simulink model "doa_debug_diego"  (R2013b) (continued)
== system doa_debug_diego == (continued)
      settings: AttributesFormatString=Latency = %<latency>
      mask Display:
      | port_label('input', 1, 'd');
      | port_label('output', 1, 'q');
      | disp(['Z^-', num2str(latency)]);
  pipeline65 : Subsystem  AncestorBlock=casper_library_delays/pipeline  mask(pipeline){latency=2}
      mask help:
      | An explicitly laid-out delay line to avoid mapping to SRL16s
      | for use in pipelining to help achieve timing closure.
      mask init:
      | pipeline_init(gcb,'latency',latency);
      settings: AttributesFormatString=Latency = %<latency>
      mask Display:
      | port_label('input', 1, 'd');
      | port_label('output', 1, 'q');
      | disp(['Z^-', num2str(latency)]);
  pipeline66 : Subsystem  AncestorBlock=casper_library_delays/pipeline  mask(pipeline){latency=2}
      mask help:
      | An explicitly laid-out delay line to avoid mapping to SRL16s
      | for use in pipelining to help achieve timing closure.
      mask init:
      | pipeline_init(gcb,'latency',latency);
      settings: AttributesFormatString=Latency = %<latency>
      mask Display:
      | port_label('input', 1, 'd');
      | port_label('output', 1, 'q');
      | disp(['Z^-', num2str(latency)]);
  pipeline67 : Subsystem  AncestorBlock=casper_library_delays/pipeline  mask(pipeline){latency=1}
      mask help:
      | An explicitly laid-out delay line to avoid mapping to SRL16s
      | for use in pipelining to help achieve timing closure.
      mask init:
      | pipeline_init(gcb,'latency',latency);
      settings: AttributesFormatString=Latency = %<latency>
      mask Display:
      | port_label('input', 1, 'd');
      | port_label('output', 1, 'q');
      | disp(['Z^-', num2str(latency)]);
  pipeline68 : Subsystem  AncestorBlock=casper_library_delays/pipeline  mask(pipeline){latency=1}
      mask help:
      | An explicitly laid-out delay line to avoid mapping to SRL16s
      | for use in pipelining to help achieve timing closure.
      mask init:
      | pipeline_init(gcb,'latency',latency);
      settings: AttributesFormatString=Latency = %<latency>
      mask Display:
      | port_label('input', 1, 'd');
      | port_label('output', 1, 'q');
      | disp(['Z^-', num2str(latency)]);
  pipeline69 : Subsystem  AncestorBlock=casper_library_delays/pipeline  mask(pipeline){latency=2}
      mask help:
      | An explicitly laid-out delay line to avoid mapping to SRL16s
      | for use in pipelining to help achieve timing closure.
      mask init:
      | pipeline_init(gcb,'latency',latency);
      settings: AttributesFormatString=Latency = %<latency>
      mask Display:
      | port_label('input', 1, 'd');
      | port_label('output', 1, 'q');
      | disp(['Z^-', num2str(latency)]);
  pipeline70 : Subsystem  AncestorBlock=casper_library_delays/pipeline  mask(pipeline){latency=2}
      mask help:
      | An explicitly laid-out delay line to avoid mapping to SRL16s
      | for use in pipelining to help achieve timing closure.
      mask init:
      | pipeline_init(gcb,'latency',latency);
      settings: AttributesFormatString=Latency = %<latency>
      mask Display:
      | port_label('input', 1, 'd');
      | port_label('output', 1, 'q');
      | disp(['Z^-', num2str(latency)]);
  pipeline71 : Subsystem  AncestorBlock=casper_library_delays/pipeline  mask(pipeline){latency=1}
      mask help:
      | An explicitly laid-out delay line to avoid mapping to SRL16s
      | for use in pipelining to help achieve timing closure.
      mask init:
      | pipeline_init(gcb,'latency',latency);
      settings: AttributesFormatString=Latency = %<latency>
      mask Display:
      | port_label('input', 1, 'd');
      | port_label('output', 1, 'q');
      | disp(['Z^-', num2str(latency)]);
  pipeline72 : Subsystem  AncestorBlock=casper_library_delays/pipeline  mask(pipeline){latency=5}
      mask help:
      | An explicitly laid-out delay line to avoid mapping to SRL16s
      | for use in pipelining to help achieve timing closure.
      mask init:
      | pipeline_init(gcb,'latency',latency);
      settings: AttributesFormatString=Latency = %<latency>
      mask Display:
      | port_label('input', 1, 'd');
      | port_label('output', 1, 'q');
      | disp(['Z^-', num2str(latency)]);
  pipeline73 : Subsystem  AncestorBlock=casper_library_delays/pipeline  mask(pipeline){latency=1}
      mask help:
      | An explicitly laid-out delay line to avoid mapping to SRL16s
      | for use in pipelining to help achieve timing closure.
      mask init:
      | pipeline_init(gcb,'latency',latency);
      settings: AttributesFormatString=Latency = %<latency>
      mask Display:
      | port_label('input', 1, 'd');
      | port_label('output', 1, 'q');
      | disp(['Z^-', num2str(latency)]);
  pipeline74 : Subsystem  AncestorBlock=casper_library_delays/pipeline  mask(pipeline){latency=2}
      mask help:
      | An explicitly laid-out delay line to avoid mapping to SRL16s
      | for use in pipelining to help achieve timing closure.
      mask init:
      | pipeline_init(gcb,'latency',latency);
      settings: AttributesFormatString=Latency = %<latency>
      mask Display:
      | port_label('input', 1, 'd');
      | port_label('output', 1, 'q');
      | disp(['Z^-', num2str(latency)]);
  pipeline75 : Subsystem  AncestorBlock=casper_library_delays/pipeline  mask(pipeline){latency=2}
      mask help:
      | An explicitly laid-out delay line to avoid mapping to SRL16s
      | for use in pipelining to help achieve timing closure.
      mask init:
      | pipeline_init(gcb,'latency',latency);
      settings: AttributesFormatString=Latency = %<latency>
      mask Display:
      | port_label('input', 1, 'd');
      | port_label('output', 1, 'q');
      | disp(['Z^-', num2str(latency)]);
  pipeline76 : Subsystem  AncestorBlock=casper_library_delays/pipeline  mask(pipeline){latency=1}
      mask help:
      | An explicitly laid-out delay line to avoid mapping to SRL16s
      | for use in pipelining to help achieve timing closure.
      mask init:
      | pipeline_init(gcb,'latency',latency);
      settings: AttributesFormatString=Latency = %<latency>
      mask Display:
      | port_label('input', 1, 'd');
      | port_label('output', 1, 'q');
      | disp(['Z^-', num2str(latency)]);
  pipeline77 : Subsystem  AncestorBlock=casper_library_delays/pipeline  mask(pipeline){latency=1}
      mask help:
      | An explicitly laid-out delay line to avoid mapping to SRL16s
      | for use in pipelining to help achieve timing closure.
      mask init:
      | pipeline_init(gcb,'latency',latency);
      settings: AttributesFormatString=Latency = %<latency>
      mask Display:
      | port_label('input', 1, 'd');
      | port_label('output', 1, 'q');
      | disp(['Z^-', num2str(latency)]);
  pipeline78 : Subsystem  AncestorBlock=casper_library_delays/pipeline  mask(pipeline){latency=1}
      mask help:
      | An explicitly laid-out delay line to avoid mapping to SRL16s
      | for use in pipelining to help achieve timing closure.
      mask init:
      | pipeline_init(gcb,'latency',latency);
      settings: AttributesFormatString=Latency = %<latency>
      mask Display:
      | port_label('input', 1, 'd');
      | port_label('output', 1, 'q');
      | disp(['Z^-', num2str(latency)]);
  pipeline79 : Subsystem  AncestorBlock=casper_library_delays/pipeline  mask(pipeline){latency=2}
      mask help:
      | An explicitly laid-out delay line to avoid mapping to SRL16s
      | for use in pipelining to help achieve timing closure.
      mask init:
      | pipeline_init(gcb,'latency',latency);
      settings: AttributesFormatString=Latency = %<latency>
      mask Display:
      | port_label('input', 1, 'd');
      | port_label('output', 1, 'q');
      | disp(['Z^-', num2str(latency)]);
  pipeline8 : Subsystem  AncestorBlock=casper_library_delays/pipeline  mask(pipeline){latency=9}
      mask help:
      | An explicitly laid-out delay line to avoid mapping to SRL16s
      | for use in pipelining to help achieve timing closure.
      mask init:
      | pipeline_init(gcb,'latency',latency);
      settings: AttributesFormatString=Latency = %<latency>
      mask Display:
      | port_label('input', 1, 'd');
      | port_label('output', 1, 'q');
      | disp(['Z^-', num2str(latency)]);
  pipeline80 : Subsystem  AncestorBlock=casper_library_delays/pipeline  mask(pipeline){latency=2}
      mask help:
      | An explicitly laid-out delay line to avoid mapping to SRL16s
      | for use in pipelining to help achieve timing closure.
      mask init:
      | pipeline_init(gcb,'latency',latency);
      settings: AttributesFormatString=Latency = %<latency>
      mask Display:
      | port_label('input', 1, 'd');
      | port_label('output', 1, 'q');
      | disp(['Z^-', num2str(latency)]);
  pipeline81 : Subsystem  AncestorBlock=casper_library_delays/pipeline  mask(pipeline){latency=1}
      mask help:
      | An explicitly laid-out delay line to avoid mapping to SRL16s
      | for use in pipelining to help achieve timing closure.
      mask init:
      | pipeline_init(gcb,'latency',latency);
      settings: AttributesFormatString=Latency = %<latency>
      mask Display:
      | port_label('input', 1, 'd');
      | port_label('output', 1, 'q');
      | disp(['Z^-', num2str(latency)]);
  pipeline82 : Subsystem  AncestorBlock=casper_library_delays/pipeline  mask(pipeline){latency=1}
      mask help:
      | An explicitly laid-out delay line to avoid mapping to SRL16s
      | for use in pipelining to help achieve timing closure.
      mask init:
      | pipeline_init(gcb,'latency',latency);
      settings: AttributesFormatString=Latency = %<latency>
      mask Display:
      | port_label('input', 1, 'd');
      | port_label('output', 1, 'q');
      | disp(['Z^-', num2str(latency)]);
  pipeline83 : Subsystem  AncestorBlock=casper_library_delays/pipeline  mask(pipeline){latency=2}
      mask help:
      | An explicitly laid-out delay line to avoid mapping to SRL16s
      | for use in pipelining to help achieve timing closure.
      mask init:
      | pipeline_init(gcb,'latency',latency);
      settings: AttributesFormatString=Latency = %<latency>
      mask Display:
      | port_label('input', 1, 'd');
      | port_label('output', 1, 'q');
      | disp(['Z^-', num2str(latency)]);
  pipeline84 : Subsystem  AncestorBlock=casper_library_delays/pipeline  mask(pipeline){latency=2}
      mask help:
      | An explicitly laid-out delay line to avoid mapping to SRL16s
      | for use in pipelining to help achieve timing closure.
      mask init:
      | pipeline_init(gcb,'latency',latency);
      settings: AttributesFormatString=Latency = %<latency>
      mask Display:
      | port_label('input', 1, 'd');
      | port_label('output', 1, 'q');
      | disp(['Z^-', num2str(latency)]);
  pipeline85 : Subsystem  AncestorBlock=casper_library_delays/pipeline  mask(pipeline){latency=1}
      mask help:
      | An explicitly laid-out delay line to avoid mapping to SRL16s
      | for use in pipelining to help achieve timing closure.
      mask init:
      | pipeline_init(gcb,'latency',latency);
      settings: AttributesFormatString=Latency = %<latency>
      mask Display:
      | port_label('input', 1, 'd');
      | port_label('output', 1, 'q');
      | disp(['Z^-', num2str(latency)]);
  pipeline86 : Subsystem  AncestorBlock=casper_library_delays/pipeline  mask(pipeline){latency=1}
      mask help:
      | An explicitly laid-out delay line to avoid mapping to SRL16s
      | for use in pipelining to help achieve timing closure.
      mask init:
      | pipeline_init(gcb,'latency',latency);
      settings: AttributesFormatString=Latency = %<latency>
      mask Display:
      | port_label('input', 1, 'd');
      | port_label('output', 1, 'q');
      | disp(['Z^-', num2str(latency)]);
  pipeline87 : Subsystem  AncestorBlock=casper_library_delays/pipeline  mask(pipeline){latency=2}
      mask help:
      | An explicitly laid-out delay line to avoid mapping to SRL16s
      | for use in pipelining to help achieve timing closure.
      mask init:
      | pipeline_init(gcb,'latency',latency);
      settings: AttributesFormatString=Latency = %<latency>
      mask Display:
      | port_label('input', 1, 'd');
      | port_label('output', 1, 'q');
      | disp(['Z^-', num2str(latency)]);
  pipeline88 : Subsystem  AncestorBlock=casper_library_delays/pipeline  mask(pipeline){latency=2}
      mask help:
      | An explicitly laid-out delay line to avoid mapping to SRL16s
      | for use in pipelining to help achieve timing closure.
      mask init:
      | pipeline_init(gcb,'latency',latency);
      settings: AttributesFormatString=Latency = %<latency>
      mask Display:
      | port_label('input', 1, 'd');
      | port_label('output', 1, 'q');
      | disp(['Z^-', num2str(latency)]);
  pipeline89 : Subsystem  AncestorBlock=casper_library_delays/pipeline  mask(pipeline){latency=1}
      mask help:
      | An explicitly laid-out delay line to avoid mapping to SRL16s
      | for use in pipelining to help achieve timing closure.
      mask init:
      | pipeline_init(gcb,'latency',latency);
      settings: AttributesFormatString=Latency = %<latency>
      mask Display:
      | port_label('input', 1, 'd');
      | port_label('output', 1, 'q');
      | disp(['Z^-', num2str(latency)]);
  pipeline90 : Subsystem  AncestorBlock=casper_library_delays/pipeline  mask(pipeline){latency=5}
      mask help:
      | An explicitly laid-out delay line to avoid mapping to SRL16s
      | for use in pipelining to help achieve timing closure.
      mask init:
      | pipeline_init(gcb,'latency',latency);
      settings: AttributesFormatString=Latency = %<latency>
      mask Display:
      | port_label('input', 1, 'd');
      | port_label('output', 1, 'q');
      | disp(['Z^-', num2str(latency)]);
  pipeline91 : Subsystem  AncestorBlock=casper_library_delays/pipeline  mask(pipeline){latency=1}
      mask help:
      | An explicitly laid-out delay line to avoid mapping to SRL16s
      | for use in pipelining to help achieve timing closure.
      mask init:
      | pipeline_init(gcb,'latency',latency);
      settings: AttributesFormatString=Latency = %<latency>
      mask Display:
      | port_label('input', 1, 'd');
      | port_label('output', 1, 'q');
      | disp(['Z^-', num2str(latency)]);
  pipeline92 : Subsystem  AncestorBlock=casper_library_delays/pipeline  mask(pipeline){latency=2}
      mask help:
      | An explicitly laid-out delay line to avoid mapping to SRL16s
      | for use in pipelining to help achieve timing closure.
      mask init:
      | pipeline_init(gcb,'latency',latency);
      settings: AttributesFormatString=Latency = %<latency>
      mask Display:
      | port_label('input', 1, 'd');
      | port_label('output', 1, 'q');
      | disp(['Z^-', num2str(latency)]);
  power{,1..7} (8 alike) : casper_library_misc/power  BitWidth=18  add_latency=2  mult_latency=3
  pulse_ext : casper_library_misc/pulse_ext  pulse_len=512
  red0_0 : Subsystem  Tag=xps:bram  AncestorBlock=xps_library/Shared_BRAM  mask{arith_type=Unsigned, addr_width=10, data_width=32, reg_prim_output=off, reg_core_output=off, optimization=Minimum_Area, data_bin_pt=0, init_vals=[0:2^10-1], sample_rate=1}
      mask prompts: arith_type "Output Data Type"; addr_width "Address width"; data_width "Data Width"; reg_prim_output "Register Primitive Output"; reg_core_output "Register Core Output"; data_bin_pt "Data Binary Point"; init_vals "Initial values (simulation only)"; sample_rate "Sample rate"
      mask init:
      | shared_bram_mask;
      mask TypeOptions arith_type: Unsigned | Signed  (2's comp)
      mask TypeOptions data_width: 8 | 16 | 32 | 64 | 128
      mask TypeOptions optimization: Minimum_Area | Low_Power
  red0_1 : Subsystem  Tag=xps:bram  AncestorBlock=xps_library/Shared_BRAM  mask{arith_type=Unsigned, addr_width=10, data_width=32, reg_prim_output=off, reg_core_output=off, optimization=Minimum_Area, data_bin_pt=0, init_vals=[0:2^10-1], sample_rate=1}
      mask prompts: arith_type "Output Data Type"; addr_width "Address width"; data_width "Data Width"; reg_prim_output "Register Primitive Output"; reg_core_output "Register Core Output"; data_bin_pt "Data Binary Point"; init_vals "Initial values (simulation only)"; sample_rate "Sample rate"
      mask init:
      | shared_bram_mask;
      mask TypeOptions arith_type: Unsigned | Signed  (2's comp)
      mask TypeOptions data_width: 8 | 16 | 32 | 64 | 128
      mask TypeOptions optimization: Minimum_Area | Low_Power
  red0_2 : Subsystem  Tag=xps:bram  AncestorBlock=xps_library/Shared_BRAM  mask{arith_type=Unsigned, addr_width=10, data_width=32, reg_prim_output=off, reg_core_output=off, optimization=Minimum_Area, data_bin_pt=0, init_vals=[0:2^10-1], sample_rate=1}
      mask prompts: arith_type "Output Data Type"; addr_width "Address width"; data_width "Data Width"; reg_prim_output "Register Primitive Output"; reg_core_output "Register Core Output"; data_bin_pt "Data Binary Point"; init_vals "Initial values (simulation only)"; sample_rate "Sample rate"
      mask init:
      | shared_bram_mask;
      mask TypeOptions arith_type: Unsigned | Signed  (2's comp)
      mask TypeOptions data_width: 8 | 16 | 32 | 64 | 128
      mask TypeOptions optimization: Minimum_Area | Low_Power
  red0_3 : Subsystem  Tag=xps:bram  AncestorBlock=xps_library/Shared_BRAM  mask{arith_type=Unsigned, addr_width=10, data_width=32, reg_prim_output=off, reg_core_output=off, optimization=Minimum_Area, data_bin_pt=0, init_vals=[0:2^10-1], sample_rate=1}
      mask prompts: arith_type "Output Data Type"; addr_width "Address width"; data_width "Data Width"; reg_prim_output "Register Primitive Output"; reg_core_output "Register Core Output"; data_bin_pt "Data Binary Point"; init_vals "Initial values (simulation only)"; sample_rate "Sample rate"
      mask init:
      | shared_bram_mask;
      mask TypeOptions arith_type: Unsigned | Signed  (2's comp)
      mask TypeOptions data_width: 8 | 16 | 32 | 64 | 128
      mask TypeOptions optimization: Minimum_Area | Low_Power
  red1_0 : Subsystem  Tag=xps:bram  AncestorBlock=xps_library/Shared_BRAM  mask{arith_type=Unsigned, addr_width=10, data_width=32, reg_prim_output=off, reg_core_output=off, optimization=Minimum_Area, data_bin_pt=0, init_vals=[0:2^10-1], sample_rate=1}
      mask prompts: arith_type "Output Data Type"; addr_width "Address width"; data_width "Data Width"; reg_prim_output "Register Primitive Output"; reg_core_output "Register Core Output"; data_bin_pt "Data Binary Point"; init_vals "Initial values (simulation only)"; sample_rate "Sample rate"
      mask init:
      | shared_bram_mask;
      mask TypeOptions arith_type: Unsigned | Signed  (2's comp)
      mask TypeOptions data_width: 8 | 16 | 32 | 64 | 128
      mask TypeOptions optimization: Minimum_Area | Low_Power
  red1_1 : Subsystem  Tag=xps:bram  AncestorBlock=xps_library/Shared_BRAM  mask{arith_type=Unsigned, addr_width=10, data_width=32, reg_prim_output=off, reg_core_output=off, optimization=Minimum_Area, data_bin_pt=0, init_vals=[0:2^10-1], sample_rate=1}
      mask prompts: arith_type "Output Data Type"; addr_width "Address width"; data_width "Data Width"; reg_prim_output "Register Primitive Output"; reg_core_output "Register Core Output"; data_bin_pt "Data Binary Point"; init_vals "Initial values (simulation only)"; sample_rate "Sample rate"
      mask init:
      | shared_bram_mask;
      mask TypeOptions arith_type: Unsigned | Signed  (2's comp)
      mask TypeOptions data_width: 8 | 16 | 32 | 64 | 128
      mask TypeOptions optimization: Minimum_Area | Low_Power
  red1_2 : Subsystem  Tag=xps:bram  AncestorBlock=xps_library/Shared_BRAM  mask{arith_type=Unsigned, addr_width=10, data_width=32, reg_prim_output=off, reg_core_output=off, optimization=Minimum_Area, data_bin_pt=0, init_vals=[0:2^10-1], sample_rate=1}
      mask prompts: arith_type "Output Data Type"; addr_width "Address width"; data_width "Data Width"; reg_prim_output "Register Primitive Output"; reg_core_output "Register Core Output"; data_bin_pt "Data Binary Point"; init_vals "Initial values (simulation only)"; sample_rate "Sample rate"
      mask init:
      | shared_bram_mask;
      mask TypeOptions arith_type: Unsigned | Signed  (2's comp)
      mask TypeOptions data_width: 8 | 16 | 32 | 64 | 128
      mask TypeOptions optimization: Minimum_Area | Low_Power
  red1_3 : Subsystem  Tag=xps:bram  AncestorBlock=xps_library/Shared_BRAM  mask{arith_type=Unsigned, addr_width=10, data_width=32, reg_prim_output=off, reg_core_output=off, optimization=Minimum_Area, data_bin_pt=0, init_vals=[0:2^10-1], sample_rate=1}
      mask prompts: arith_type "Output Data Type"; addr_width "Address width"; data_width "Data Width"; reg_prim_output "Register Primitive Output"; reg_core_output "Register Core Output"; data_bin_pt "Data Binary Point"; init_vals "Initial values (simulation only)"; sample_rate "Sample rate"
      mask init:
      | shared_bram_mask;
      mask TypeOptions arith_type: Unsigned | Signed  (2's comp)
      mask TypeOptions data_width: 8 | 16 | 32 | 64 | 128
      mask TypeOptions optimization: Minimum_Area | Low_Power
  sig_acc : Subsystem
  simple_bram_vacc_0a_0 : Subsystem  AncestorBlock=casper_library_accumulators/simple_bram_vacc  mask(simple_bram_vacc){vec_len=512, arith_type=Unsigned, n_bits=64, bin_pt=35}
      mask prompts: vec_len "Vector length:"; arith_type "Output type:"; n_bits "Bit width (output):"; bin_pt "Binary point (output):"
      mask help: This simple vector accumulator is based on a shift register. It outputs its previous accumulated vector after a new_acc pulse is received.
      mask init:
      | simple_bram_vacc_init(gcb, ...
      |     'vec_len', vec_len, ...
      |     'arith_type', arith_type, ...
      |     'n_bits', n_bits, ...
      |     'bin_pt', bin_pt);
      mask TypeOptions arith_type: Signed | Unsigned
  simple_bram_vacc_0a_1 : Subsystem  AncestorBlock=casper_library_accumulators/simple_bram_vacc  mask(simple_bram_vacc){vec_len=512, arith_type=Unsigned, n_bits=64, bin_pt=35}
      mask prompts: vec_len "Vector length:"; arith_type "Output type:"; n_bits "Bit width (output):"; bin_pt "Binary point (output):"
      mask help: This simple vector accumulator is based on a shift register. It outputs its previous accumulated vector after a new_acc pulse is received.
      mask init:
      | simple_bram_vacc_init(gcb, ...
      |     'vec_len', vec_len, ...
      |     'arith_type', arith_type, ...
      |     'n_bits', n_bits, ...
      |     'bin_pt', bin_pt);
      mask TypeOptions arith_type: Signed | Unsigned
  simple_bram_vacc_0a_2 : Subsystem  AncestorBlock=casper_library_accumulators/simple_bram_vacc  mask(simple_bram_vacc){vec_len=512, arith_type=Unsigned, n_bits=64, bin_pt=35}
      mask prompts: vec_len "Vector length:"; arith_type "Output type:"; n_bits "Bit width (output):"; bin_pt "Binary point (output):"
      mask help: This simple vector accumulator is based on a shift register. It outputs its previous accumulated vector after a new_acc pulse is received.
      mask init:
      | simple_bram_vacc_init(gcb, ...
      |     'vec_len', vec_len, ...
      |     'arith_type', arith_type, ...
      |     'n_bits', n_bits, ...
      |     'bin_pt', bin_pt);
      mask TypeOptions arith_type: Signed | Unsigned
  simple_bram_vacc_0a_3 : Subsystem  AncestorBlock=casper_library_accumulators/simple_bram_vacc  mask(simple_bram_vacc){vec_len=512, arith_type=Unsigned, n_bits=64, bin_pt=35}
      mask prompts: vec_len "Vector length:"; arith_type "Output type:"; n_bits "Bit width (output):"; bin_pt "Binary point (output):"
      mask help: This simple vector accumulator is based on a shift register. It outputs its previous accumulated vector after a new_acc pulse is received.
      mask init:
      | simple_bram_vacc_init(gcb, ...
      |     'vec_len', vec_len, ...
      |     'arith_type', arith_type, ...
      |     'n_bits', n_bits, ...
      |     'bin_pt', bin_pt);
      mask TypeOptions arith_type: Signed | Unsigned
  simple_bram_vacc_0c_0 : Subsystem  AncestorBlock=casper_library_accumulators/simple_bram_vacc  mask(simple_bram_vacc){vec_len=512, arith_type=Unsigned, n_bits=64, bin_pt=35}
      mask prompts: vec_len "Vector length:"; arith_type "Output type:"; n_bits "Bit width (output):"; bin_pt "Binary point (output):"
      mask help: This simple vector accumulator is based on a shift register. It outputs its previous accumulated vector after a new_acc pulse is received.
      mask init:
      | simple_bram_vacc_init(gcb, ...
      |     'vec_len', vec_len, ...
      |     'arith_type', arith_type, ...
      |     'n_bits', n_bits, ...
      |     'bin_pt', bin_pt);
      mask TypeOptions arith_type: Signed | Unsigned
  simple_bram_vacc_0c_1 : Subsystem  AncestorBlock=casper_library_accumulators/simple_bram_vacc  mask(simple_bram_vacc){vec_len=512, arith_type=Unsigned, n_bits=64, bin_pt=35}
      mask prompts: vec_len "Vector length:"; arith_type "Output type:"; n_bits "Bit width (output):"; bin_pt "Binary point (output):"
      mask help: This simple vector accumulator is based on a shift register. It outputs its previous accumulated vector after a new_acc pulse is received.
      mask init:
      | simple_bram_vacc_init(gcb, ...
      |     'vec_len', vec_len, ...
      |     'arith_type', arith_type, ...
      |     'n_bits', n_bits, ...
      |     'bin_pt', bin_pt);
      mask TypeOptions arith_type: Signed | Unsigned
  simple_bram_vacc_0c_2 : Subsystem  AncestorBlock=casper_library_accumulators/simple_bram_vacc  mask(simple_bram_vacc){vec_len=512, arith_type=Unsigned, n_bits=64, bin_pt=35}
      mask prompts: vec_len "Vector length:"; arith_type "Output type:"; n_bits "Bit width (output):"; bin_pt "Binary point (output):"
      mask help: This simple vector accumulator is based on a shift register. It outputs its previous accumulated vector after a new_acc pulse is received.
      mask init:
      | simple_bram_vacc_init(gcb, ...
      |     'vec_len', vec_len, ...
      |     'arith_type', arith_type, ...
      |     'n_bits', n_bits, ...
      |     'bin_pt', bin_pt);
      mask TypeOptions arith_type: Signed | Unsigned
  simple_bram_vacc_0c_3 : Subsystem  AncestorBlock=casper_library_accumulators/simple_bram_vacc  mask(simple_bram_vacc){vec_len=512, arith_type=Unsigned, n_bits=64, bin_pt=35}
      mask prompts: vec_len "Vector length:"; arith_type "Output type:"; n_bits "Bit width (output):"; bin_pt "Binary point (output):"
      mask help: This simple vector accumulator is based on a shift register. It outputs its previous accumulated vector after a new_acc pulse is received.
      mask init:
      | simple_bram_vacc_init(gcb, ...
      |     'vec_len', vec_len, ...
      |     'arith_type', arith_type, ...
      |     'n_bits', n_bits, ...
      |     'bin_pt', bin_pt);
      mask TypeOptions arith_type: Signed | Unsigned
  sync_gen : Subsystem  AncestorBlock=casper_library_misc/sync_gen  mask(sync_gen){sim_acc_len=1, fft_size=4096, fft_simult_inputs=8, pfb_fir_taps=4, reorder_vec=[2 2 9], scale=1}
      mask prompts: sim_acc_len "Simulation Accumulation Length"; fft_size "FFT Size"; fft_simult_inputs "Simultaneous Inputs (FFT)"; pfb_fir_taps "Taps in pfb_fir (use 1 if no pfb_fir)"; reorder_vec "Reorder Orders (e.g. [2 2 2])"
      mask help:
      | This block computes the sync period based on system parameters as
      | described in Casper memo.  Detailed descritions of the block's
      | variables can be found in the 'Block Documentation' section of
      | the CASPER wiki.
      mask init:
      | sync_gen_init(gcb, ...
      |     'sim_acc_len', sim_acc_len, ...
      |     'fft_size', fft_size, ...
      |     'fft_simult_inputs', fft_simult_inputs,...
      |     'pfb_fir_taps', pfb_fir_taps, ...
      |     'reorder_vec', reorder_vec, ...
      |     'scale', scale);
      settings: AttributesFormatString=sim_sync_period=36864
      mask Help:
      | SyncPeriod=LCM(reorder_vec)*(acc_num)*(pfb_fir_len)*(fft_size/fft_simult_inputs)
      | 
      | See CASPER sync pulse memo for more details.
  unsig_acc : Subsystem
  unsig_acc1 : Subsystem
  x11_0 : Subsystem  Tag=xps:bram  AncestorBlock=xps_library/Shared_BRAM  mask{arith_type=Unsigned, addr_width=9, data_width=64, reg_prim_output=off, reg_core_output=off, optimization=Minimum_Area, data_bin_pt=0, init_vals=[0:2^10-1], sample_rate=1}
      mask prompts: arith_type "Output Data Type"; addr_width "Address width"; data_width "Data Width"; reg_prim_output "Register Primitive Output"; reg_core_output "Register Core Output"; data_bin_pt "Data Binary Point"; init_vals "Initial values (simulation only)"; sample_rate "Sample rate"
      mask init:
      | shared_bram_mask;
      mask TypeOptions arith_type: Unsigned | Signed  (2's comp)
      mask TypeOptions data_width: 8 | 16 | 32 | 64 | 128
      mask TypeOptions optimization: Minimum_Area | Low_Power
  x11_1 : Subsystem  Tag=xps:bram  AncestorBlock=xps_library/Shared_BRAM  mask{arith_type=Unsigned, addr_width=9, data_width=64, reg_prim_output=off, reg_core_output=off, optimization=Minimum_Area, data_bin_pt=0, init_vals=[0:2^10-1], sample_rate=1}
      mask prompts: arith_type "Output Data Type"; addr_width "Address width"; data_width "Data Width"; reg_prim_output "Register Primitive Output"; reg_core_output "Register Core Output"; data_bin_pt "Data Binary Point"; init_vals "Initial values (simulation only)"; sample_rate "Sample rate"
      mask init:
      | shared_bram_mask;
      mask TypeOptions arith_type: Unsigned | Signed  (2's comp)
      mask TypeOptions data_width: 8 | 16 | 32 | 64 | 128
      mask TypeOptions optimization: Minimum_Area | Low_Power
  x12_0 : Subsystem  Tag=xps:bram  AncestorBlock=xps_library/Shared_BRAM  mask{arith_type=Unsigned, addr_width=9, data_width=64, reg_prim_output=off, reg_core_output=off, optimization=Minimum_Area, data_bin_pt=0, init_vals=[0:2^10-1], sample_rate=1}
      mask prompts: arith_type "Output Data Type"; addr_width "Address width"; data_width "Data Width"; reg_prim_output "Register Primitive Output"; reg_core_output "Register Core Output"; data_bin_pt "Data Binary Point"; init_vals "Initial values (simulation only)"; sample_rate "Sample rate"
      mask init:
      | shared_bram_mask;
      mask TypeOptions arith_type: Unsigned | Signed  (2's comp)
      mask TypeOptions data_width: 8 | 16 | 32 | 64 | 128
      mask TypeOptions optimization: Minimum_Area | Low_Power
  x12_1 : Subsystem  Tag=xps:bram  AncestorBlock=xps_library/Shared_BRAM  mask{arith_type=Unsigned, addr_width=9, data_width=64, reg_prim_output=off, reg_core_output=off, optimization=Minimum_Area, data_bin_pt=0, init_vals=[0:2^10-1], sample_rate=1}
      mask prompts: arith_type "Output Data Type"; addr_width "Address width"; data_width "Data Width"; reg_prim_output "Register Primitive Output"; reg_core_output "Register Core Output"; data_bin_pt "Data Binary Point"; init_vals "Initial values (simulation only)"; sample_rate "Sample rate"
      mask init:
      | shared_bram_mask;
      mask TypeOptions arith_type: Unsigned | Signed  (2's comp)
      mask TypeOptions data_width: 8 | 16 | 32 | 64 | 128
      mask TypeOptions optimization: Minimum_Area | Low_Power
  x22_0 : Subsystem  Tag=xps:bram  AncestorBlock=xps_library/Shared_BRAM  mask{arith_type=Unsigned, addr_width=9, data_width=64, reg_prim_output=off, reg_core_output=off, optimization=Minimum_Area, data_bin_pt=0, init_vals=[0:2^10-1], sample_rate=1}
      mask prompts: arith_type "Output Data Type"; addr_width "Address width"; data_width "Data Width"; reg_prim_output "Register Primitive Output"; reg_core_output "Register Core Output"; data_bin_pt "Data Binary Point"; init_vals "Initial values (simulation only)"; sample_rate "Sample rate"
      mask init:
      | shared_bram_mask;
      mask TypeOptions arith_type: Unsigned | Signed  (2's comp)
      mask TypeOptions data_width: 8 | 16 | 32 | 64 | 128
      mask TypeOptions optimization: Minimum_Area | Low_Power
  x22_1 : Subsystem  Tag=xps:bram  AncestorBlock=xps_library/Shared_BRAM  mask{arith_type=Unsigned, addr_width=9, data_width=64, reg_prim_output=off, reg_core_output=off, optimization=Minimum_Area, data_bin_pt=0, init_vals=[0:2^10-1], sample_rate=1}
      mask prompts: arith_type "Output Data Type"; addr_width "Address width"; data_width "Data Width"; reg_prim_output "Register Primitive Output"; reg_core_output "Register Core Output"; data_bin_pt "Data Binary Point"; init_vals "Initial values (simulation only)"; sample_rate "Sample rate"
      mask init:
      | shared_bram_mask;
      mask TypeOptions arith_type: Unsigned | Signed  (2's comp)
      mask TypeOptions data_width: 8 | 16 | 32 | 64 | 128
      mask TypeOptions optimization: Minimum_Area | Low_Power
  xr11_acc : Subsystem  Tag=xps:bram  AncestorBlock=xps_library/Shared_BRAM  mask{arith_type=Unsigned, addr_width=10, data_width=64, reg_prim_output=off, reg_core_output=off, optimization=Minimum_Area, data_bin_pt=0, init_vals=[0:2^10-1], sample_rate=1}
      mask prompts: arith_type "Output Data Type"; addr_width "Address width"; data_width "Data Width"; reg_prim_output "Register Primitive Output"; reg_core_output "Register Core Output"; data_bin_pt "Data Binary Point"; init_vals "Initial values (simulation only)"; sample_rate "Sample rate"
      mask init:
      | shared_bram_mask;
      mask TypeOptions arith_type: Unsigned | Signed  (2's comp)
      mask TypeOptions data_width: 8 | 16 | 32 | 64 | 128
      mask TypeOptions optimization: Minimum_Area | Low_Power
  xr12_acc : Subsystem  Tag=xps:bram  AncestorBlock=xps_library/Shared_BRAM  mask{arith_type=Unsigned, addr_width=10, data_width=64, reg_prim_output=off, reg_core_output=off, optimization=Minimum_Area, data_bin_pt=0, init_vals=[0:2^10-1], sample_rate=1}
      mask prompts: arith_type "Output Data Type"; addr_width "Address width"; data_width "Data Width"; reg_prim_output "Register Primitive Output"; reg_core_output "Register Core Output"; data_bin_pt "Data Binary Point"; init_vals "Initial values (simulation only)"; sample_rate "Sample rate"
      mask init:
      | shared_bram_mask;
      mask TypeOptions arith_type: Unsigned | Signed  (2's comp)
      mask TypeOptions data_width: 8 | 16 | 32 | 64 | 128
      mask TypeOptions optimization: Minimum_Area | Low_Power
  xr22_acc : Subsystem  Tag=xps:bram  AncestorBlock=xps_library/Shared_BRAM  mask{arith_type=Unsigned, addr_width=10, data_width=64, reg_prim_output=off, reg_core_output=off, optimization=Minimum_Area, data_bin_pt=0, init_vals=[0:2^10-1], sample_rate=1}
      mask prompts: arith_type "Output Data Type"; addr_width "Address width"; data_width "Data Width"; reg_prim_output "Register Primitive Output"; reg_core_output "Register Core Output"; data_bin_pt "Data Binary Point"; init_vals "Initial values (simulation only)"; sample_rate "Sample rate"
      mask init:
      | shared_bram_mask;
      mask TypeOptions arith_type: Unsigned | Signed  (2's comp)
      mask TypeOptions data_width: 8 | 16 | 32 | 64 | 128
      mask TypeOptions optimization: Minimum_Area | Low_Power
  wires:
  Constant1/1 -> fft_wideband_real0/2(shift)
  fft_wideband_real0/6(of) -> Terminator1/1
  bus_create/1(bus_out) -> pipeline276/1
  acc_len/1(in_reg) -> pipeline250/1(d)
  cnt_rst/1(in_reg) -> pipeline251/1(d)
  pipeline250/1(q) -> [acc_len]
  pipeline251/1(q) -> Slice1/1
  Band-Limited White Noise/1 -> asiaa_adc5g0/1(sim_a)
  Constant13/1 -> asiaa_adc5g0/3(sim_sync)
  sync_gen/1(sync_out) -> [sync_out]
  pipeline262/1 -> pfb_fir_real0/8(pol1_in7)
  pipeline257/1 -> pfb_fir_real0/3(pol1_in2)
  pipeline261/1 -> pfb_fir_real0/7(pol1_in6)
  pipeline260/1 -> pfb_fir_real0/6(pol1_in5)
  pipeline259/1 -> pfb_fir_real0/5(pol1_in4)
  pipeline258/1 -> pfb_fir_real0/4(pol1_in3)
  pipeline263/1 -> pfb_fir_real0/9(pol1_in8)
  pipeline256/1 -> pfb_fir_real0/2(pol1_in1)
  pipeline16/1 -> pfb_fir_real0/1(sync)
  pfb_fir_real0/1(sync_out) -> Delay15/1
  Delay15/1 -> fft_wideband_real0/1(sync)
  fft_wideband_real0/1(sync_out) -> pipeline275/1
  fft_wideband_real0/2(out00) -> pipeline1/1
  fft_wideband_real0/3(out01) -> pipeline2/1
  fft_wideband_real0/4(out02) -> pipeline3/1
  fft_wideband_real0/5(out03) -> pipeline9/1
  pipeline276/1 -> adcsnap0/1(din)
  Constant4/1 -> adcsnap0/3(trig), adcsnap0/2(we)
  Slice1/1 -> [cnt_rst]
  Shift12/1 -> fft_wideband_real0/5(in02)
  Shift9/1 -> fft_wideband_real0/6(in03)
  Shift11/1 -> fft_wideband_real0/7(in04)
  Shift10/1 -> fft_wideband_real0/8(in05)
  Shift8/1 -> fft_wideband_real0/9(in06)
  Shift7/1 -> fft_wideband_real0/10(in07)
  Shift13/1 -> fft_wideband_real0/4(in01)
  Shift15/1 -> fft_wideband_real0/3(in00)
  pfb_fir_real0/2(pol1_out1) -> Shift15/1
  pfb_fir_real0/3(pol1_out2) -> Shift13/1
  pfb_fir_real0/4(pol1_out3) -> Shift12/1
  pfb_fir_real0/5(pol1_out4) -> Shift9/1
  pfb_fir_real0/6(pol1_out5) -> Shift11/1
  pfb_fir_real0/7(pol1_out6) -> Shift10/1
  pfb_fir_real0/8(pol1_out7) -> Shift8/1
  pfb_fir_real0/9(pol1_out8) -> Shift7/1
  Band-Limited White Noise2/1 -> asiaa_adc5g0/2(sim_c)
  asiaa_adc5g0/17(sync_out) -> Terminator/1
  asiaa_adc5g0/1(a0) -> conv_bus0/1(i0)
  asiaa_adc5g0/2(a1) -> conv_bus0/2(i1)
  asiaa_adc5g0/3(a2) -> conv_bus0/3(i2)
  asiaa_adc5g0/4(a3) -> conv_bus0/4(i3)
  asiaa_adc5g0/5(a4) -> conv_bus0/5(i4)
  asiaa_adc5g0/6(a5) -> conv_bus0/6(i5)
  asiaa_adc5g0/7(a6) -> conv_bus0/7(i6)
  asiaa_adc5g0/8(a7) -> conv_bus0/8(i7)
  conv_bus0/7(q6) -> bus_create/7(in7), pipeline262/1
  conv_bus0/5(q4) -> bus_create/5(in5), pipeline260/1
  conv_bus0/4(q3) -> bus_create/4(in4), pipeline259/1
  conv_bus0/3(q2) -> bus_create/3(in3), pipeline258/1
  conv_bus0/2(q1) -> bus_create/2(in2), pipeline257/1
  conv_bus0/1(q0) -> bus_create/1(in1), pipeline256/1
  bus_create1/1(bus_out) -> pipeline4/1
  pipeline4/1 -> adcsnap1/1(din)
  Constant8/1 -> adcsnap1/3(trig), adcsnap1/2(we)
  conv_bus0/8(q7) -> bus_create/8(in8), pipeline263/1
  [sync_out] -> pipeline16/1
  pipeline72/1(q) -> acc_cntrl0/2(sync)
  [cnt_rst] -> acc_cntrl0/3(rst)
  [acc_len] -> acc_cntrl0/1(acc_len)
  power/1 -> Delay32/1
  power1/1 -> Delay34/1
  power2/1 -> Delay35/1
  power3/1 -> Delay36/1
  dout_0a_0/1(data_out) -> Terminator9/1
  Reinterpret31/1 -> dout_0a_0/2(data_in)
  simple_bram_vacc_0a_0/1(dout) -> pipeline74/1(d)
  simple_bram_vacc_0a_0/2(valid) -> pipeline56/1(d)
  pipeline73/1(q) -> simple_bram_vacc_0a_0/2(din)
  pipeline59/1(q) -> pipeline57/1(d)
  pipeline74/1(q) -> Reinterpret31/1
  pipeline56/1(q) -> Counter1/2, dout_0a_0/3(we)
  pipeline57/1(q) -> Counter1/1
  pipeline58/1(q) -> simple_bram_vacc_0a_0/1(new_acc)
  dout_0a_1/1(data_out) -> Terminator6/1
  Reinterpret24/1 -> dout_0a_1/2(data_in)
  simple_bram_vacc_0a_1/1(dout) -> pipeline61/1(d)
  simple_bram_vacc_0a_1/2(valid) -> pipeline62/1(d)
  pipeline60/1(q) -> simple_bram_vacc_0a_1/2(din)
  pipeline61/1(q) -> Reinterpret24/1
  pipeline62/1(q) -> dout_0a_1/3(we)
  pipeline63/1(q) -> simple_bram_vacc_0a_1/1(new_acc)
  dout_0a_2/1(data_out) -> Terminator7/1
  Reinterpret25/1 -> dout_0a_2/2(data_in)
  simple_bram_vacc_0a_2/1(dout) -> pipeline65/1(d)
  simple_bram_vacc_0a_2/2(valid) -> pipeline66/1(d)
  pipeline64/1(q) -> simple_bram_vacc_0a_2/2(din)
  pipeline65/1(q) -> Reinterpret25/1
  pipeline66/1(q) -> dout_0a_2/3(we)
  pipeline67/1(q) -> simple_bram_vacc_0a_2/1(new_acc)
  dout_0a_3/1(data_out) -> Terminator8/1
  Reinterpret26/1 -> dout_0a_3/2(data_in)
  simple_bram_vacc_0a_3/1(dout) -> pipeline69/1(d)
  simple_bram_vacc_0a_3/2(valid) -> pipeline70/1(d)
  pipeline68/1(q) -> simple_bram_vacc_0a_3/2(din)
  pipeline69/1(q) -> Reinterpret26/1
  pipeline70/1(q) -> dout_0a_3/3(we)
  pipeline71/1(q) -> simple_bram_vacc_0a_3/1(new_acc)
  Delay32/1 -> pipeline73/1(d)
  Delay34/1 -> pipeline60/1(d)
  Delay35/1 -> pipeline64/1(d)
  Delay36/1 -> pipeline68/1(d)
  Counter1/1 -> dout_0a_1/1(addr), dout_0a_2/1(addr), dout_0a_3/1(addr), dout_0a_0/1(addr)
  acc_cntrl0/1(new_acc) -> pipeline63/1(d), pipeline71/1(d), pipeline67/1(d), pipeline58/1(d), pipeline59/1(d)
  pipeline275/1 -> pipeline72/1(d), [sync_fft]
  pipeline1/1 -> power/1, [adc0_ch0]
  pipeline2/1 -> [adc0_ch1], power1/1
  pipeline3/1 -> [adc0_ch2], power2/1
  pipeline9/1 -> [adc0_ch3], power3/1
  Constant10/1 -> fft_wideband_real1/2(shift)
  fft_wideband_real1/6(of) -> Terminator5/1
  pipeline18/1 -> pfb_fir_real1/8(pol1_in7)
  pipeline11/1 -> pfb_fir_real1/3(pol1_in2)
  pipeline17/1 -> pfb_fir_real1/7(pol1_in6)
  pipeline15/1 -> pfb_fir_real1/6(pol1_in5)
  pipeline14/1 -> pfb_fir_real1/5(pol1_in4)
  pipeline12/1 -> pfb_fir_real1/4(pol1_in3)
  pipeline19/1 -> pfb_fir_real1/9(pol1_in8)
  pipeline10/1 -> pfb_fir_real1/2(pol1_in1)
  pipeline6/1 -> pfb_fir_real1/1(sync)
  pfb_fir_real1/1(sync_out) -> Delay3/1
  Delay3/1 -> fft_wideband_real1/1(sync)
  fft_wideband_real1/1(sync_out) -> pipeline20/1
  fft_wideband_real1/2(out00) -> pipeline5/1
  fft_wideband_real1/3(out01) -> pipeline7/1
  fft_wideband_real1/4(out02) -> pipeline21/1
  fft_wideband_real1/5(out03) -> pipeline93/1
  Shift3/1 -> fft_wideband_real1/5(in02)
  Shift16/1 -> fft_wideband_real1/6(in03)
  Shift2/1 -> fft_wideband_real1/7(in04)
  Shift1/1 -> fft_wideband_real1/8(in05)
  Shift14/1 -> fft_wideband_real1/9(in06)
  Shift6/1 -> fft_wideband_real1/10(in07)
  Shift4/1 -> fft_wideband_real1/4(in01)
  Shift5/1 -> fft_wideband_real1/3(in00)
  pfb_fir_real1/2(pol1_out1) -> Shift5/1
  pfb_fir_real1/3(pol1_out2) -> Shift4/1
  pfb_fir_real1/4(pol1_out3) -> Shift3/1
  pfb_fir_real1/5(pol1_out4) -> Shift16/1
  pfb_fir_real1/6(pol1_out5) -> Shift2/1
  pfb_fir_real1/7(pol1_out6) -> Shift1/1
  pfb_fir_real1/8(pol1_out7) -> Shift14/1
  pfb_fir_real1/9(pol1_out8) -> Shift6/1
  conv_bus1/7(q6) -> pipeline18/1, bus_create1/7(in7)
  conv_bus1/6(q5) -> bus_create1/6(in6), pipeline17/1
  conv_bus1/5(q4) -> bus_create1/5(in5), pipeline15/1
  conv_bus1/4(q3) -> bus_create1/4(in4), pipeline14/1
  conv_bus1/3(q2) -> bus_create1/3(in3), pipeline12/1
  conv_bus1/2(q1) -> bus_create1/2(in2), pipeline11/1
  conv_bus1/1(q0) -> bus_create1/1(in1), pipeline10/1
  conv_bus1/8(q7) -> bus_create1/8(in8), pipeline19/1
  [sync_out] -> pipeline6/1
  pipeline90/1(q) -> acc_cntrl1/2(sync)
  [cnt_rst] -> acc_cntrl1/3(rst)
  [acc_len] -> acc_cntrl1/1(acc_len)
  power4/1 -> Delay7/1
  power5/1 -> Delay8/1
  power6/1 -> Delay9/1
  power7/1 -> Delay10/1
  dout_0c_0/1(data_out) -> Terminator13/1
  Reinterpret4/1 -> dout_0c_0/2(data_in)
  simple_bram_vacc_0c_0/1(dout) -> pipeline92/1(d)
  simple_bram_vacc_0c_0/2(valid) -> pipeline22/1(d)
  pipeline91/1(q) -> simple_bram_vacc_0c_0/2(din)
  pipeline77/1(q) -> pipeline75/1(d)
  pipeline92/1(q) -> Reinterpret4/1
  pipeline22/1(q) -> dout_0c_0/3(we), Counter2/2
  pipeline75/1(q) -> Counter2/1
  pipeline76/1(q) -> simple_bram_vacc_0c_0/1(new_acc)
  dout_0c_1/1(data_out) -> Terminator10/1
  Reinterpret1/1 -> dout_0c_1/2(data_in)
  simple_bram_vacc_0c_1/1(dout) -> pipeline79/1(d)
  simple_bram_vacc_0c_1/2(valid) -> pipeline80/1(d)
  pipeline78/1(q) -> simple_bram_vacc_0c_1/2(din)
  pipeline79/1(q) -> Reinterpret1/1
  pipeline80/1(q) -> dout_0c_1/3(we)
  pipeline81/1(q) -> simple_bram_vacc_0c_1/1(new_acc)
  dout_0c_2/1(data_out) -> Terminator11/1
  Reinterpret2/1 -> dout_0c_2/2(data_in)
  simple_bram_vacc_0c_2/1(dout) -> pipeline83/1(d)
  simple_bram_vacc_0c_2/2(valid) -> pipeline84/1(d)
  pipeline82/1(q) -> simple_bram_vacc_0c_2/2(din)
  pipeline83/1(q) -> Reinterpret2/1
  pipeline84/1(q) -> dout_0c_2/3(we)
  pipeline85/1(q) -> simple_bram_vacc_0c_2/1(new_acc)
  dout_0c_3/1(data_out) -> Terminator12/1
  Reinterpret3/1 -> dout_0c_3/2(data_in)
  simple_bram_vacc_0c_3/1(dout) -> pipeline87/1(d)
  simple_bram_vacc_0c_3/2(valid) -> pipeline88/1(d)
  pipeline86/1(q) -> simple_bram_vacc_0c_3/2(din)
  pipeline87/1(q) -> Reinterpret3/1
  pipeline88/1(q) -> dout_0c_3/3(we)
  pipeline89/1(q) -> simple_bram_vacc_0c_3/1(new_acc)
  Delay7/1 -> pipeline91/1(d)
  Delay8/1 -> pipeline78/1(d)
  Delay9/1 -> pipeline82/1(d)
  Delay10/1 -> pipeline86/1(d)
  Counter2/1 -> dout_0c_0/1(addr), dout_0c_1/1(addr), dout_0c_2/1(addr), dout_0c_3/1(addr)
  acc_cntrl1/1(new_acc) -> pipeline77/1(d), pipeline76/1(d), pipeline85/1(d), pipeline89/1(d), pipeline81/1(d)
  pipeline20/1 -> pipeline90/1(d)
  pipeline5/1 -> [adc1_ch0], power4/1
  pipeline7/1 -> power5/1, [adc1_ch1]
  pipeline21/1 -> [adc1_ch2], power6/1
  pipeline93/1 -> [adc1_ch3], power7/1
  asiaa_adc5g0/12(c3) -> conv_bus1/4(i3)
  asiaa_adc5g0/14(c5) -> conv_bus1/6(i5)
  asiaa_adc5g0/10(c1) -> conv_bus1/2(i1)
  asiaa_adc5g0/13(c4) -> conv_bus1/5(i4)
  asiaa_adc5g0/9(c0) -> conv_bus1/1(i0)
  asiaa_adc5g0/15(c6) -> conv_bus1/7(i6)
  asiaa_adc5g0/16(c7) -> conv_bus1/8(i7)
  asiaa_adc5g0/11(c2) -> conv_bus1/3(i2)
  conv_bus0/6(q5) -> bus_create/6(in6), pipeline261/1
  corr_seba/5(r11_ch3) -> pipeline189/1(d)
  corr_seba/2(r11_ch0) -> pipeline186/1(d)
  corr_seba/4(r11_ch2) -> pipeline188/1(d)
  corr_seba/3(r11_ch1) -> pipeline187/1(d)
  corr_seba/6(r12_ch0) -> pipeline190/1(d)
  corr_seba/7(r12_ch1) -> pipeline191/1(d)
  corr_seba/8(r12_ch2) -> pipeline192/1(d)
  corr_seba/9(r12_ch3) -> pipeline193/1(d)
  corr_seba/10(r22_ch0) -> pipeline194/1(d)
  corr_seba/13(r22_ch3) -> pipeline197/1(d)
  corr_seba/12(r22_ch2) -> pipeline196/1(d)
  corr_seba/11(r22_ch1) -> pipeline195/1(d)
  centrosym/1(sync_out) -> pipeline155/1(d)
  centrosym/2(x1_ch0) -> pipeline156/1(d)
  centrosym/3(x2_ch0) -> pipeline157/1(d)
  centrosym/4(x1_ch1) -> pipeline158/1(d)
  centrosym/5(x2_ch1) -> pipeline159/1(d)
  centrosym/6(x1_ch2) -> pipeline160/1(d)
  centrosym/7(x2_ch2) -> pipeline161/1(d)
  centrosym/8(x1_ch3) -> pipeline162/1(d)
  centrosym/9(x2_ch3) -> pipeline163/1(d)
  pipeline8/1(q) -> edge_detect/1(in), Accumulator2/3, Accumulator1/3, Accumulator/3
  edge_detect/1(out) -> Accumulator2/2, Accumulator1/2, Accumulator/2, Delay/1, Register5/2, Register4/2, Register3/2
  unsig_acc1/8(valid3) -> pipeline211/1(d)
  unsig_acc/1(acc0) -> pipeline198/1(d)
  unsig_acc/3(acc1) -> pipeline200/1(d)
  unsig_acc/5(acc2) -> pipeline201/1(d)
  unsig_acc/7(acc3) -> pipeline202/1(d)
  add_chanels/1(Out1) -> Accumulator/1
  sig_acc/1(acc0) -> pipeline203/1(d)
  sig_acc/3(acc1) -> pipeline204/1(d)
  sig_acc/5(acc2) -> pipeline205/1(d)
  sig_acc/7(acc3) -> pipeline206/1(d)
  unsig_acc1/1(acc0) -> pipeline207/1(d)
  unsig_acc1/3(acc1) -> pipeline208/1(d)
  unsig_acc1/5(acc2) -> pipeline209/1(d)
  unsig_acc1/7(acc3) -> pipeline210/1(d)
  add_chanels1/1(Out1) -> Accumulator1/1
  add_chanels2/1(Out1) -> Accumulator2/1
  unsig_acc/2(valid0) -> pipeline199/1(d)
  unsig_acc/4(valid1) -> Terminator22/1
  unsig_acc/6(valid2) -> Terminator24/1
  unsig_acc/8(valid3) -> Terminator25/1
  sig_acc/2(valid0) -> Terminator26/1
  sig_acc/4(valid1) -> Terminator27/1
  sig_acc/6(valid2) -> Terminator28/1
  sig_acc/8(valid3) -> Terminator29/1
  unsig_acc1/2(valid0) -> Terminator30/1
  unsig_acc1/4(valid1) -> Terminator31/1
  unsig_acc1/6(valid2) -> Terminator23/1
  Accumulator2/1 -> Register3/1
  Accumulator1/1 -> Register4/1
  Accumulator/1 -> Register5/1
  Concat1/1 -> x11_1/2(data_in)
  Concat2/1 -> x12_0/2(data_in)
  Concat3/1 -> x12_1/2(data_in)
  Concat4/1 -> x22_0/2(data_in)
  Concat5/1 -> x22_1/2(data_in)
  Counter/1 -> x22_1/1(addr), x22_0/1(addr), x12_1/1(addr), x12_0/1(addr), x11_1/1(addr), x11_0/1(addr)
  pipeline23/1(q) -> acc_cntrl4/2(sync)
  [cnt_rst] -> acc_cntrl4/3(rst)
  [acc_len] -> acc_cntrl4/1(acc_len)
  acc_cntrl4/1(new_acc) -> centrosym_diego/1(sync_in), centrosym/1(sync_in)
  Concat/1 -> x11_0/2(data_in)
  corr_seba/1(sync_out) -> pipeline185/1(d)
  pipeline38/1(q) -> pipeline116/1(d)
  pipeline116/1(q) -> centrosym_diego/2(c0_ch0), centrosym/2(c0_ch0)
  pipeline133/1(q) -> pipeline132/1(d)
  pipeline132/1(q) -> centrosym_diego/3(c0_ch1), centrosym/3(c0_ch1)
  pipeline135/1(q) -> pipeline134/1(d)
  pipeline134/1(q) -> centrosym_diego/4(c0_ch2), centrosym/4(c0_ch2)
  pipeline137/1(q) -> pipeline136/1(d)
  pipeline136/1(q) -> centrosym/5(c0_ch3), centrosym_diego/5(c0_ch3)
  pipeline139/1(q) -> pipeline138/1(d)
  pipeline138/1(q) -> centrosym_diego/6(cx_ch0), centrosym/6(cx_ch0)
  pipeline141/1(q) -> pipeline140/1(d)
  pipeline140/1(q) -> centrosym_diego/7(cx_ch1), centrosym/7(cx_ch1)
  pipeline143/1(q) -> pipeline142/1(d)
  pipeline142/1(q) -> centrosym_diego/8(cx_ch2), centrosym/8(cx_ch2)
  pipeline145/1(q) -> pipeline144/1(d)
  pipeline144/1(q) -> centrosym_diego/9(cx_ch3), centrosym/9(cx_ch3)
  pipeline13/1(q) -> complex_convert/1(in), pipeline38/1(d)
  pipeline25/1(q) -> complex_convert1/1(in), pipeline133/1(d)
  pipeline24/1(q) -> complex_convert2/1(in), pipeline135/1(d)
  pipeline27/1(q) -> complex_convert3/1(in), pipeline137/1(d)
  pipeline26/1(q) -> complex_convert4/1(in), pipeline139/1(d)
  pipeline29/1(q) -> complex_convert5/1(in), pipeline141/1(d)
  pipeline28/1(q) -> complex_convert6/1(in), pipeline143/1(d)
  pipeline30/1(q) -> complex_convert7/1(in), pipeline145/1(d)
  pipeline154/1(q) -> pipeline/1, pipeline23/1(d)
  pipeline155/1(q) -> corr_seba/1(sync_in)
  pipeline156/1(q) -> corr_seba/2(x1_ch0)
  pipeline158/1(q) -> corr_seba/4(x1_ch1)
  pipeline157/1(q) -> corr_seba/3(x2_ch0)
  pipeline159/1(q) -> corr_seba/5(x2_ch1)
  pipeline162/1(q) -> corr_seba/8(x1_ch3)
  pipeline160/1(q) -> corr_seba/6(x1_ch2)
  pipeline161/1(q) -> corr_seba/7(x2_ch2)
  pipeline163/1(q) -> corr_seba/9(x2_ch3)
  pipeline185/1(q) -> pipeline212/1(d), unsig_acc1/1(new_acc), sig_acc/1(new_acc), unsig_acc/1(new_acc)
  pipeline186/1(q) -> unsig_acc/2(ch0)
  pipeline188/1(q) -> unsig_acc/4(ch2)
  pipeline187/1(q) -> unsig_acc/3(ch1)
  pipeline192/1(q) -> sig_acc/4(ch2)
  pipeline190/1(q) -> sig_acc/2(ch0)
  pipeline189/1(q) -> unsig_acc/5(ch3)
  pipeline191/1(q) -> sig_acc/3(ch1)
  pipeline196/1(q) -> unsig_acc1/4(ch2)
  pipeline194/1(q) -> unsig_acc1/2(ch0)
  pipeline195/1(q) -> unsig_acc1/3(ch1)
  pipeline193/1(q) -> sig_acc/5(ch3)
  pipeline197/1(q) -> unsig_acc1/5(ch3)
  Register5/1 -> Delay4/1
  Register4/1 -> Delay5/1
  Register3/1 -> Delay16/1
  Reinterpret16/1 -> Concat2/1
  Reinterpret17/1 -> Concat2/2
  Reinterpret18/1 -> Concat3/1
  Reinterpret19/1 -> Concat3/2
  Reinterpret23/1 -> Concat1/2
  Reinterpret22/1 -> Concat1/1
  Reinterpret21/1 -> Concat/2
  Reinterpret20/1 -> Concat/1
  Reinterpret27/1 -> Concat4/1
  Reinterpret28/1 -> Concat4/2
  Reinterpret29/1 -> Concat5/1
  Reinterpret30/1 -> Concat5/2
  pipeline198/1(q) -> pipeline244/1(d), pipeline266/1(d)
  pipeline199/1(q) -> pipeline243/1(d)
  pipeline200/1(q) -> pipeline245/1(d), pipeline267/1(d)
  pipeline201/1(q) -> pipeline246/1(d), pipeline268/1(d)
  pipeline202/1(q) -> pipeline247/1(d), pipeline269/1(d)
  pipeline203/1(q) -> pipeline248/1(d), pipeline270/1(d)
  pipeline204/1(q) -> pipeline249/1(d), pipeline271/1(d)
  pipeline205/1(q) -> pipeline252/1(d), pipeline272/1(d)
  pipeline206/1(q) -> pipeline253/1(d), pipeline273/1(d)
  pipeline207/1(q) -> pipeline254/1(d), pipeline274/1(d)
  pipeline208/1(q) -> pipeline255/1(d), pipeline277/1(d)
  pipeline209/1(q) -> pipeline264/1(d), pipeline278/1(d)
  pipeline210/1(q) -> pipeline279/1(d), pipeline265/1(d)
  pipeline211/1(q) -> pipeline280/1(d)
  pipeline212/1(q) -> pipeline242/1(d)
  pipeline242/1(q) -> Counter/1
  pipeline243/1(q) -> Counter/2, x22_1/3(we), x22_0/3(we), x12_1/3(we), x12_0/3(we), x11_1/3(we), x11_0/3(we)
  pipeline244/1(q) -> Reinterpret20/1
  pipeline245/1(q) -> Reinterpret21/1
  pipeline246/1(q) -> Reinterpret22/1
  pipeline247/1(q) -> Reinterpret23/1
  pipeline248/1(q) -> Reinterpret16/1
  pipeline253/1(q) -> Reinterpret19/1
  pipeline252/1(q) -> Reinterpret18/1
  pipeline249/1(q) -> Reinterpret17/1
  pipeline255/1(q) -> Reinterpret28/1
  pipeline254/1(q) -> Reinterpret27/1
  pipeline264/1(q) -> Reinterpret29/1
  pipeline265/1(q) -> Reinterpret30/1
  pipeline269/1(q) -> add_chanels/4(ch3)
  pipeline267/1(q) -> add_chanels/2(ch1)
  pipeline268/1(q) -> add_chanels/3(ch2)
  pipeline266/1(q) -> add_chanels/1(ch0)
  pipeline272/1(q) -> add_chanels1/3(ch2)
  pipeline270/1(q) -> add_chanels1/1(ch0)
  pipeline271/1(q) -> add_chanels1/2(ch1)
  pipeline273/1(q) -> add_chanels1/4(ch3)
  pipeline278/1(q) -> add_chanels2/3(ch2)
  pipeline279/1(q) -> add_chanels2/4(ch3)
  pipeline274/1(q) -> add_chanels2/1(ch0)
  pipeline277/1(q) -> add_chanels2/2(ch1)
  pipeline280/1(q) -> pipeline8/1(d)
  Reinterpret40/1 -> xr11_acc/2(data_in)
  Reinterpret41/1 -> xr12_acc/2(data_in)
  Reinterpret42/1 -> xr22_acc/2(data_in)
  Delay/1 -> xr12_acc/3(we), xr22_acc/3(we), xr11_acc/3(we), Counter6/1
  Delay4/1 -> Reinterpret40/1
  Delay5/1 -> Reinterpret41/1
  Delay16/1 -> Reinterpret42/1
  Counter6/1 -> xr12_acc/1(addr), xr22_acc/1(addr), xr11_acc/1(addr)
  gain/1(in_reg) -> Subsystem2/1(gain)
  [adc0_ch0] -> Subsystem2/3(din0)
  [sync_fft] -> Subsystem2/2(sync)
  [adc0_ch1] -> Subsystem2/4(din1)
  [adc0_ch2] -> Subsystem2/5(din2)
  [adc0_ch3] -> Subsystem2/6(din3)
  [adc1_ch0] -> Subsystem2/7(din4)
  [adc1_ch1] -> Subsystem2/8(din5)
  [adc1_ch2] -> Subsystem2/9(din6)
  [adc1_ch3] -> Subsystem2/10(din7)
  Subsystem2/1(sync_out) -> pipeline154/1(d)
  Subsystem2/2(Out1) -> pipeline13/1(d)
  Subsystem2/3(Out2) -> pipeline25/1(d)
  Subsystem2/4(Out3) -> pipeline24/1(d)
  Subsystem2/5(Out4) -> pipeline27/1(d)
  Subsystem2/6(Out5) -> pipeline26/1(d)
  Subsystem2/7(Out6) -> pipeline29/1(d)
  Subsystem2/8(Out7) -> pipeline28/1(d)
  Subsystem2/9(Out8) -> pipeline30/1(d)
  pipeline/1 -> Counter3/1, pulse_ext/1
  pulse_ext/1 -> Delay1/1
  Delay1/1 -> red0_0/3(we), red0_1/3(we), red0_2/3(we), red0_3/3(we), red1_0/3(we), red1_1/3(we), red1_3/3(we), red1_2/3(we), Counter3/2
  Counter3/1 -> red0_1/1(addr), red1_2/1(addr), red1_3/1(addr), red1_1/1(addr), red1_0/1(addr), red0_3/1(addr), red0_2/1(addr), red0_0/1(addr)
  complex_convert/1(out) -> red0_0/2(data_in)
  complex_convert1/1(out) -> red0_1/2(data_in)
  complex_convert2/1(out) -> red0_2/2(data_in)
  complex_convert3/1(out) -> red0_3/2(data_in)
  complex_convert4/1(out) -> red1_0/2(data_in)
  complex_convert5/1(out) -> red1_1/2(data_in)
  complex_convert6/1(out) -> red1_2/2(data_in)
  complex_convert7/1(out) -> red1_3/2(data_in)
  centrosym_diego/2(x1_ch0) -> pipeline32/1(d)
  centrosym_diego/7(x2_ch1) -> pipeline37/1(d)
  centrosym_diego/4(x1_ch2) -> pipeline34/1(d)
  centrosym_diego/6(x2_ch0) -> pipeline36/1(d)
  centrosym_diego/5(x1_ch3) -> pipeline35/1(d)
  centrosym_diego/8(x2_ch2) -> pipeline39/1(d)
  centrosym_diego/9(x2_ch3) -> pipeline40/1(d)
  centrosym_diego/3(x1_ch1) -> pipeline33/1(d)
  centrosym_diego/1(sync_out) -> pipeline31/1(d)
  pipeline31/1(q) -> corrs_diego/1(sync_in)
  pipeline32/1(q) -> corrs_diego/2(x1_ch0)
  pipeline33/1(q) -> corrs_diego/3(x1_ch1)
  pipeline34/1(q) -> corrs_diego/4(x1_ch2)
  pipeline37/1(q) -> corrs_diego/7(x2_ch1)
  pipeline39/1(q) -> corrs_diego/8(x2_ch2)
  pipeline35/1(q) -> corrs_diego/5(x1_ch3)
  pipeline36/1(q) -> corrs_diego/6(x2_ch0)
  pipeline40/1(q) -> corrs_diego/9(x2_ch3)
